simulink model "wlan_phy_rx_pmd"  (Simulink 7.8)
settings: Solver=VariableStepDiscrete  SolverMode=SingleTasking  StartTime=0.0  StopTime=simtime  RelTol=1e-3
PostLoadFcn:
  | wlan_phy_rx_init
InitFcn:
  | wlan_phy_rx_init
StartFcn:
  | wlan_phy_rx_init
values from neighbouring files (symbols this model uses):
  barker_seq20 = 0.95 * circshift(barker_seq20, [0 4]) ./ max(abs(barker_seq20))    [wlan_phy_rx_init.m]
  bit_scrambler_lfsr_bytes = bi2de(reshape(repmat(scr, 1, 8), 8, 127)', 'left-msb')    [wlan_phy_rx_init.m]
  deinterleave_ROM = [deinterleave_ROM interleave_64QAM zeros(1, 512-length(interleave_64QAM))]    [wlan_phy_rx_init.m]
  longCorr_coef_i = [3*real(LTS_corr)]    [wlan_phy_rx_init.m]
  longCorr_coef_q = [3*imag(LTS_corr)]    [wlan_phy_rx_init.m]
  MAX_CP_LEN = 32    [wlan_phy_rx_init.m]
  MAX_NCBPS = 288    [wlan_phy_rx_init.m]
  MAX_NUM_BYTES = 4096    [wlan_phy_rx_init.m]
  MAX_NUM_SC = 64    [wlan_phy_rx_init.m]
  MAX_NUM_SYMS = 600    [wlan_phy_rx_init.m]
  n_bits_preFFT_sampBuff = ceil(log2(4*MAX_NUM_SC))    [wlan_phy_rx_init.m]
  NCBPS_16QAM = 192    [wlan_phy_rx_init.m]
  NCBPS_64QAM = 288    [wlan_phy_rx_init.m]
  NCBPS_BPSK = 48    [wlan_phy_rx_init.m]
  NCBPS_QPSK = 96    [wlan_phy_rx_init.m]
  NDBPS_16QAM12 = 96    [wlan_phy_rx_init.m]
  NDBPS_16QAM34 = 144    [wlan_phy_rx_init.m]
  NDBPS_64QAM23 = 192    [wlan_phy_rx_init.m]
  NDBPS_64QAM34 = 216    [wlan_phy_rx_init.m]
  NDBPS_BPSK12 = 24    [wlan_phy_rx_init.m]
  NDBPS_BPSK34 = 36    [wlan_phy_rx_init.m]
  NDBPS_QPSK12 = 48    [wlan_phy_rx_init.m]
  NDBPS_QPSK34 = 72    [wlan_phy_rx_init.m]
  paylod_vec_samp_time = 8    [wlan_phy_rx_init.m]
  PHY_MIN_PKT_LEN = 14    [wlan_phy_rx_init.m]
  scr_ind_rev = zeros(1,128)    [wlan_phy_rx_init.m]
undefined symbols (not defined in the file or in its folder): gcbh, xlBlockLoadCallback, xlGetNormalizedPeriod, xlipgetpartsetting
libraries: xbsIndex_r4 x3519, simulink x1, xbsEDKLib_r4 x1
(part 6 of 21)

== system wlan_phy_rx_pmd/Inputs/RF A Inputs ==
  Rst : Inport
  IQ Valid : Inport  Port=2
  Constant2 : Constant  Value=2
  Constant4 : Constant  Value=5
  Delay1 : xbsIndex_r4/Delay  rst=off  infoeditControl=Selection of Reset will increase slice count due to use of real FFs and instead of SRLs  en=off  latency=1  dbl_ovrd=off  reg_retiming=off  block_type=delay
  Delay2 : xbsIndex_r4/Delay  rst=off  infoeditControl=Selection of Reset will increase slice count due to use of real FFs and instead of SRLs  en=off  latency=1  dbl_ovrd=off  reg_retiming=off  block_type=delay
  Down Sample : xbsIndex_r4/Down Sample  sample_ratio=16  sample_phase="Last Value of Frame  (most efficient)"  en=off  latency=1  dbl_ovrd=off  block_type=dsamp
  From Workspace : FromWorkspace  VariableName=raw_rx_I  SampleTime=paylod_vec_samp_time  Interpolate=off  OutputAfterFinalValue=Setting to zero
  From Workspace1 : FromWorkspace  VariableName=raw_rx_Q  SampleTime=paylod_vec_samp_time  Interpolate=off  OutputAfterFinalValue=Setting to zero
  From Workspace2 : FromWorkspace  VariableName=samps_rssi_avg  SampleTime=paylod_vec_samp_time  Interpolate=off  OutputAfterFinalValue=Setting to zero
  RF A RSSI Sum : Subsystem
  RFA_G_BB : xbsIndex_r4/Gateway In  gui_display_data_type=Fixed-point  arith_type=Unsigned  n_bits=5  bin_pt=0  preci_type=Single  exp_width=8  frac_width=24  quantization="Round  (unbiased: +/- Inf)"  overflow=Saturate  period=1  dbl_ovrd=off  timing_constraint=None  locs_specified=off  LOCs={}  UseAsADC=off  ADCChannel='1'  inherit_from_input=off  hdl_port=on  block_type=gatewayin
  RFA_G_RF : xbsIndex_r4/Gateway In  gui_display_data_type=Fixed-point  arith_type=Unsigned  n_bits=2  bin_pt=0  preci_type=Single  exp_width=8  frac_width=24  quantization="Round  (unbiased: +/- Inf)"  overflow=Saturate  period=1  dbl_ovrd=off  timing_constraint=None  locs_specified=off  LOCs={}  UseAsADC=off  ADCChannel='1'  inherit_from_input=off  hdl_port=on  block_type=gatewayin
  RFA_RSSI : xbsIndex_r4/Gateway In  gui_display_data_type=Fixed-point  arith_type=Unsigned  n_bits=10  bin_pt=0  preci_type=Single  exp_width=8  frac_width=24  quantization="Round  (unbiased: +/- Inf)"  overflow=Saturate  period=1  dbl_ovrd=off  timing_constraint=None  locs_specified=off  LOCs={}  UseAsADC=off  ADCChannel='1'  inherit_from_input=off  hdl_port=on  block_type=gatewayin
  RFA_RX_I : xbsIndex_r4/Gateway In  gui_display_data_type=Fixed-point  arith_type="Signed  (2's comp)"  n_bits=16  bin_pt=15  preci_type=Single  exp_width=8  frac_width=24  quantization="Round  (unbiased: +/- Inf)"  overflow=Saturate  period=1  dbl_ovrd=off  timing_constraint=None  locs_specified=off  LOCs={}  UseAsADC=off  ADCChannel='1'  inherit_from_input=off  hdl_port=on  block_type=gatewayin
  RFA_RX_Q : xbsIndex_r4/Gateway In  gui_display_data_type=Fixed-point  arith_type="Signed  (2's comp)"  n_bits=16  bin_pt=15  preci_type=Single  exp_width=8  frac_width=24  quantization="Round  (unbiased: +/- Inf)"  overflow=Saturate  period=1  dbl_ovrd=off  timing_constraint=None  locs_specified=off  LOCs={}  UseAsADC=off  ADCChannel='1'  inherit_from_input=off  hdl_port=on  block_type=gatewayin
  Register : xbsIndex_r4/Register  init=0  rst=on  en=on  dbl_ovrd=off  block_type=register
  Register1 : xbsIndex_r4/Register  init=0  rst=on  en=on  dbl_ovrd=off  block_type=register
  Register2 : xbsIndex_r4/Register  init=0  rst=off  en=off  dbl_ovrd=off  block_type=register
  Register3 : xbsIndex_r4/Register  init=0  rst=on  en=off  dbl_ovrd=off  block_type=register
  Register4 : xbsIndex_r4/Register  init=0  rst=off  en=off  dbl_ovrd=off  block_type=register
  Register5 : xbsIndex_r4/Register  init=0  rst=off  en=off  dbl_ovrd=off  block_type=register
  ADC I : Outport
  ADC Q : Outport  Port=2
  Avg RSSI : Outport  Port=3
  tag scope: CS_ADC_I=global, CS_ADC_Q=global, CS_G_BB=global, CS_G_RF=global, CS_RFA_RSSI=global, RFA_AGC_G_BB=global, RFA_AGC_G_RF=global
  wires:
  From Workspace/1 -> RFA_RX_I/1
  From Workspace1/1 -> RFA_RX_Q/1
  RFA_RX_I/1 -> Register2/1
  RFA_RX_Q/1 -> Register4/1
  Register/1 -> ADC I/1
  Register1/1 -> ADC Q/1
  Register3/1 -> Down Sample/1
  Down Sample/1 -> RF A RSSI Sum/1(RSSI)
  RF A RSSI Sum/1(Sum) -> [CS_RFA_RSSI], Avg RSSI/1
  RFA_RSSI/1 -> Register5/1
  Register2/1 -> Register/1, [CS_ADC_I]
  Register4/1 -> Register1/1, [CS_ADC_Q]
  Register5/1 -> Register3/1
  Rst/1 -> Register/2, Register1/2, Register3/2
  IQ Valid/1 -> Register1/3, Register/3
  RFA_G_RF/1 -> Delay1/1
  RFA_G_BB/1 -> Delay2/1
  Delay1/1 -> [CS_G_RF], [RFA_AGC_G_RF]
  Delay2/1 -> [CS_G_BB], [RFA_AGC_G_BB]
  Constant2/1 -> RFA_G_RF/1
  Constant4/1 -> RFA_G_BB/1

== system wlan_phy_rx_pmd/Inputs/RF B Inputs ==
  Rst : Inport
  IQ Valid : Inport  Port=2
  Constant6 : Constant  Value=2
  Constant7 : Constant  Value=5
  Delay4 : xbsIndex_r4/Delay  rst=off  infoeditControl=Selection of Reset will increase slice count due to use of real FFs and instead of SRLs  en=off  latency=1  dbl_ovrd=off  reg_retiming=off  block_type=delay
  Delay5 : xbsIndex_r4/Delay  rst=off  infoeditControl=Selection of Reset will increase slice count due to use of real FFs and instead of SRLs  en=off  latency=1  dbl_ovrd=off  reg_retiming=off  block_type=delay
  Down Sample : xbsIndex_r4/Down Sample  sample_ratio=16  sample_phase="Last Value of Frame  (most efficient)"  en=off  latency=1  dbl_ovrd=off  block_type=dsamp
  From Workspace : FromWorkspace  VariableName=raw_rx_I  SampleTime=paylod_vec_samp_time  Interpolate=off  OutputAfterFinalValue=Setting to zero
  From Workspace1 : FromWorkspace  VariableName=raw_rx_Q  SampleTime=paylod_vec_samp_time  Interpolate=off  OutputAfterFinalValue=Setting to zero
  From Workspace2 : FromWorkspace  VariableName=samps_rssi_avg  SampleTime=paylod_vec_samp_time  Interpolate=off  OutputAfterFinalValue=Setting to zero
  RF B RSSI Sum : Subsystem
  RFB_G_BB : xbsIndex_r4/Gateway In  gui_display_data_type=Fixed-point  arith_type=Unsigned  n_bits=5  bin_pt=0  preci_type=Single  exp_width=8  frac_width=24  quantization="Round  (unbiased: +/- Inf)"  overflow=Saturate  period=1  dbl_ovrd=off  timing_constraint=None  locs_specified=off  LOCs={}  UseAsADC=off  ADCChannel='1'  inherit_from_input=off  hdl_port=on  block_type=gatewayin
  RFB_G_RF : xbsIndex_r4/Gateway In  gui_display_data_type=Fixed-point  arith_type=Unsigned  n_bits=2  bin_pt=0  preci_type=Single  exp_width=8  frac_width=24  quantization="Round  (unbiased: +/- Inf)"  overflow=Saturate  period=1  dbl_ovrd=off  timing_constraint=None  locs_specified=off  LOCs={}  UseAsADC=off  ADCChannel='1'  inherit_from_input=off  hdl_port=on  block_type=gatewayin
  RFB_RSSI : xbsIndex_r4/Gateway In  gui_display_data_type=Fixed-point  arith_type=Unsigned  n_bits=10  bin_pt=0  preci_type=Single  exp_width=8  frac_width=24  quantization="Round  (unbiased: +/- Inf)"  overflow=Saturate  period=1  dbl_ovrd=off  timing_constraint=None  locs_specified=off  LOCs={}  UseAsADC=off  ADCChannel='1'  inherit_from_input=off  hdl_port=on  block_type=gatewayin
  RFB_RX_I : xbsIndex_r4/Gateway In  gui_display_data_type=Fixed-point  arith_type="Signed  (2's comp)"  n_bits=16  bin_pt=15  preci_type=Single  exp_width=8  frac_width=24  quantization="Round  (unbiased: +/- Inf)"  overflow=Saturate  period=1  dbl_ovrd=off  timing_constraint=None  locs_specified=off  LOCs={}  UseAsADC=off  ADCChannel='1'  inherit_from_input=off  hdl_port=on  block_type=gatewayin
  RFB_RX_Q : xbsIndex_r4/Gateway In  gui_display_data_type=Fixed-point  arith_type="Signed  (2's comp)"  n_bits=16  bin_pt=15  preci_type=Single  exp_width=8  frac_width=24  quantization="Round  (unbiased: +/- Inf)"  overflow=Saturate  period=1  dbl_ovrd=off  timing_constraint=None  locs_specified=off  LOCs={}  UseAsADC=off  ADCChannel='1'  inherit_from_input=off  hdl_port=on  block_type=gatewayin
  Register : xbsIndex_r4/Register  init=0  rst=on  en=on  dbl_ovrd=off  block_type=register
  Register1 : xbsIndex_r4/Register  init=0  rst=on  en=on  dbl_ovrd=off  block_type=register
  Register2 : xbsIndex_r4/Register  init=0  rst=off  en=off  dbl_ovrd=off  block_type=register
  Register3 : xbsIndex_r4/Register  init=0  rst=on  en=off  dbl_ovrd=off  block_type=register
  Register4 : xbsIndex_r4/Register  init=0  rst=off  en=off  dbl_ovrd=off  block_type=register
  Register5 : xbsIndex_r4/Register  init=0  rst=off  en=off  dbl_ovrd=off  block_type=register
  ADC I : Outport
  ADC Q : Outport  Port=2
  Avg RSSI : Outport  Port=3
  tag scope: RFB_AGC_G_BB=global, RFB_AGC_G_RF=global
  wires:
  IQ Valid/1 -> Register/3, Register1/3
  Rst/1 -> Register3/2, Register1/2, Register/2
  Register5/1 -> Register3/1
  Register4/1 -> Register1/1
  Register2/1 -> Register/1
  RFB_RSSI/1 -> Register5/1
  RF B RSSI Sum/1(Sum) -> Avg RSSI/1
  Down Sample/1 -> RF B RSSI Sum/1(RSSI)
  Register3/1 -> Down Sample/1
  Register1/1 -> ADC Q/1
  Register/1 -> ADC I/1
  RFB_RX_Q/1 -> Register4/1
  RFB_RX_I/1 -> Register2/1
  RFB_G_RF/1 -> Delay4/1
  RFB_G_BB/1 -> Delay5/1
  Delay4/1 -> [RFB_AGC_G_RF]
  Delay5/1 -> [RFB_AGC_G_BB]
  Constant6/1 -> RFB_G_RF/1
  Constant7/1 -> RFB_G_BB/1

== system wlan_phy_rx_pmd/Inputs/RF C Inputs ==
  Rst : Inport
  IQ Valid : Inport  Port=2
  Constant2 : Constant  Value=2
  Constant4 : Constant  Value=5
  Delay1 : xbsIndex_r4/Delay  rst=off  infoeditControl=Selection of Reset will increase slice count due to use of real FFs and instead of SRLs  en=off  latency=1  dbl_ovrd=off  reg_retiming=off  block_type=delay
  Delay2 : xbsIndex_r4/Delay  rst=off  infoeditControl=Selection of Reset will increase slice count due to use of real FFs and instead of SRLs  en=off  latency=1  dbl_ovrd=off  reg_retiming=off  block_type=delay
  Down Sample : xbsIndex_r4/Down Sample  sample_ratio=16  sample_phase="Last Value of Frame  (most efficient)"  en=off  latency=1  dbl_ovrd=off  block_type=dsamp
  From Workspace : FromWorkspace  VariableName=raw_rx_I  SampleTime=paylod_vec_samp_time  Interpolate=off  OutputAfterFinalValue=Setting to zero
  From Workspace1 : FromWorkspace  VariableName=raw_rx_Q  SampleTime=paylod_vec_samp_time  Interpolate=off  OutputAfterFinalValue=Setting to zero
  From Workspace2 : FromWorkspace  VariableName=samps_rssi_avg  SampleTime=paylod_vec_samp_time  Interpolate=off  OutputAfterFinalValue=Setting to zero
  RF C RSSI Sum : Subsystem
  RFC_G_BB : xbsIndex_r4/Gateway In  gui_display_data_type=Fixed-point  arith_type=Unsigned  n_bits=5  bin_pt=0  preci_type=Single  exp_width=8  frac_width=24  quantization="Round  (unbiased: +/- Inf)"  overflow=Saturate  period=1  dbl_ovrd=off  timing_constraint=None  locs_specified=off  LOCs={}  UseAsADC=off  ADCChannel='1'  inherit_from_input=off  hdl_port=on  block_type=gatewayin
  RFC_G_RF : xbsIndex_r4/Gateway In  gui_display_data_type=Fixed-point  arith_type=Unsigned  n_bits=2  bin_pt=0  preci_type=Single  exp_width=8  frac_width=24  quantization="Round  (unbiased: +/- Inf)"  overflow=Saturate  period=1  dbl_ovrd=off  timing_constraint=None  locs_specified=off  LOCs={}  UseAsADC=off  ADCChannel='1'  inherit_from_input=off  hdl_port=on  block_type=gatewayin
  RFC_RSSI : xbsIndex_r4/Gateway In  gui_display_data_type=Fixed-point  arith_type=Unsigned  n_bits=10  bin_pt=0  preci_type=Single  exp_width=8  frac_width=24  quantization="Round  (unbiased: +/- Inf)"  overflow=Saturate  period=1  dbl_ovrd=off  timing_constraint=None  locs_specified=off  LOCs={}  UseAsADC=off  ADCChannel='1'  inherit_from_input=off  hdl_port=on  block_type=gatewayin
  RFC_RX_I : xbsIndex_r4/Gateway In  gui_display_data_type=Fixed-point  arith_type="Signed  (2's comp)"  n_bits=16  bin_pt=15  preci_type=Single  exp_width=8  frac_width=24  quantization="Round  (unbiased: +/- Inf)"  overflow=Saturate  period=1  dbl_ovrd=off  timing_constraint=None  locs_specified=off  LOCs={}  UseAsADC=off  ADCChannel='1'  inherit_from_input=off  hdl_port=on  block_type=gatewayin
  RFC_RX_Q : xbsIndex_r4/Gateway In  gui_display_data_type=Fixed-point  arith_type="Signed  (2's comp)"  n_bits=16  bin_pt=15  preci_type=Single  exp_width=8  frac_width=24  quantization="Round  (unbiased: +/- Inf)"  overflow=Saturate  period=1  dbl_ovrd=off  timing_constraint=None  locs_specified=off  LOCs={}  UseAsADC=off  ADCChannel='1'  inherit_from_input=off  hdl_port=on  block_type=gatewayin
  Register : xbsIndex_r4/Register  init=0  rst=on  en=on  dbl_ovrd=off  block_type=register
  Register1 : xbsIndex_r4/Register  init=0  rst=on  en=on  dbl_ovrd=off  block_type=register
  Register2 : xbsIndex_r4/Register  init=0  rst=off  en=off  dbl_ovrd=off  block_type=register
  Register3 : xbsIndex_r4/Register  init=0  rst=on  en=off  dbl_ovrd=off  block_type=register
  Register4 : xbsIndex_r4/Register  init=0  rst=off  en=off  dbl_ovrd=off  block_type=register
  Register5 : xbsIndex_r4/Register  init=0  rst=off  en=off  dbl_ovrd=off  block_type=register
  ADC I : Outport
  ADC Q : Outport  Port=2
  Avg RSSI : Outport  Port=3
  tag scope: CS_ADC_I_RFC=global, CS_ADC_Q_RFC=global, RFC_AGC_G_BB=global, RFC_AGC_G_RF=global
  wires:
  Constant4/1 -> RFC_G_BB/1
  Constant2/1 -> RFC_G_RF/1
  Delay2/1 -> [RFC_AGC_G_BB]
  Delay1/1 -> [RFC_AGC_G_RF]
  RFC_G_BB/1 -> Delay2/1
  RFC_G_RF/1 -> Delay1/1
  IQ Valid/1 -> Register/3, Register1/3
  Rst/1 -> Register3/2, Register1/2, Register/2
  Register5/1 -> Register3/1
  Register4/1 -> Register1/1, [CS_ADC_Q_RFC]
  Register2/1 -> Register/1, [CS_ADC_I_RFC]
  RFC_RSSI/1 -> Register5/1
  RF C RSSI Sum/1(Sum) -> Avg RSSI/1
  Down Sample/1 -> RF C RSSI Sum/1(RSSI)
  Register3/1 -> Down Sample/1
  Register1/1 -> ADC Q/1
  Register/1 -> ADC I/1
  RFC_RX_Q/1 -> Register4/1
  RFC_RX_I/1 -> Register2/1
  From Workspace1/1 -> RFC_RX_Q/1
  From Workspace/1 -> RFC_RX_I/1

== system wlan_phy_rx_pmd/Inputs/RF D Inputs ==
  Rst : Inport
  IQ Valid : Inport  Port=2
  Constant6 : Constant  Value=2
  Constant7 : Constant  Value=5
  Delay4 : xbsIndex_r4/Delay  rst=off  infoeditControl=Selection of Reset will increase slice count due to use of real FFs and instead of SRLs  en=off  latency=1  dbl_ovrd=off  reg_retiming=off  block_type=delay
  Delay5 : xbsIndex_r4/Delay  rst=off  infoeditControl=Selection of Reset will increase slice count due to use of real FFs and instead of SRLs  en=off  latency=1  dbl_ovrd=off  reg_retiming=off  block_type=delay
  Down Sample : xbsIndex_r4/Down Sample  sample_ratio=16  sample_phase="Last Value of Frame  (most efficient)"  en=off  latency=1  dbl_ovrd=off  block_type=dsamp
  From Workspace : FromWorkspace  VariableName=raw_rx_I  SampleTime=paylod_vec_samp_time  Interpolate=off  OutputAfterFinalValue=Setting to zero
  From Workspace1 : FromWorkspace  VariableName=raw_rx_Q  SampleTime=paylod_vec_samp_time  Interpolate=off  OutputAfterFinalValue=Setting to zero
  From Workspace2 : FromWorkspace  VariableName=samps_rssi_avg  SampleTime=paylod_vec_samp_time  Interpolate=off  OutputAfterFinalValue=Setting to zero
  RF D RSSI Sum : Subsystem
  RFD_G_BB : xbsIndex_r4/Gateway In  gui_display_data_type=Fixed-point  arith_type=Unsigned  n_bits=5  bin_pt=0  preci_type=Single  exp_width=8  frac_width=24  quantization="Round  (unbiased: +/- Inf)"  overflow=Saturate  period=1  dbl_ovrd=off  timing_constraint=None  locs_specified=off  LOCs={}  UseAsADC=off  ADCChannel='1'  inherit_from_input=off  hdl_port=on  block_type=gatewayin
  RFD_G_RF : xbsIndex_r4/Gateway In  gui_display_data_type=Fixed-point  arith_type=Unsigned  n_bits=2  bin_pt=0  preci_type=Single  exp_width=8  frac_width=24  quantization="Round  (unbiased: +/- Inf)"  overflow=Saturate  period=1  dbl_ovrd=off  timing_constraint=None  locs_specified=off  LOCs={}  UseAsADC=off  ADCChannel='1'  inherit_from_input=off  hdl_port=on  block_type=gatewayin
  RFD_RSSI : xbsIndex_r4/Gateway In  gui_display_data_type=Fixed-point  arith_type=Unsigned  n_bits=10  bin_pt=0  preci_type=Single  exp_width=8  frac_width=24  quantization="Round  (unbiased: +/- Inf)"  overflow=Saturate  period=1  dbl_ovrd=off  timing_constraint=None  locs_specified=off  LOCs={}  UseAsADC=off  ADCChannel='1'  inherit_from_input=off  hdl_port=on  block_type=gatewayin
  RFD_RX_I : xbsIndex_r4/Gateway In  gui_display_data_type=Fixed-point  arith_type="Signed  (2's comp)"  n_bits=16  bin_pt=15  preci_type=Single  exp_width=8  frac_width=24  quantization="Round  (unbiased: +/- Inf)"  overflow=Saturate  period=1  dbl_ovrd=off  timing_constraint=None  locs_specified=off  LOCs={}  UseAsADC=off  ADCChannel='1'  inherit_from_input=off  hdl_port=on  block_type=gatewayin
  RFD_RX_Q : xbsIndex_r4/Gateway In  gui_display_data_type=Fixed-point  arith_type="Signed  (2's comp)"  n_bits=16  bin_pt=15  preci_type=Single  exp_width=8  frac_width=24  quantization="Round  (unbiased: +/- Inf)"  overflow=Saturate  period=1  dbl_ovrd=off  timing_constraint=None  locs_specified=off  LOCs={}  UseAsADC=off  ADCChannel='1'  inherit_from_input=off  hdl_port=on  block_type=gatewayin
  Register : xbsIndex_r4/Register  init=0  rst=on  en=on  dbl_ovrd=off  block_type=register
  Register1 : xbsIndex_r4/Register  init=0  rst=on  en=on  dbl_ovrd=off  block_type=register
  Register2 : xbsIndex_r4/Register  init=0  rst=off  en=off  dbl_ovrd=off  block_type=register
  Register3 : xbsIndex_r4/Register  init=0  rst=on  en=off  dbl_ovrd=off  block_type=register
  Register4 : xbsIndex_r4/Register  init=0  rst=off  en=off  dbl_ovrd=off  block_type=register
  Register5 : xbsIndex_r4/Register  init=0  rst=off  en=off  dbl_ovrd=off  block_type=register
  ADC I : Outport
  ADC Q : Outport  Port=2
  Avg RSSI : Outport  Port=3
  tag scope: RFD_AGC_G_BB=global, RFD_AGC_G_RF=global
  wires:
  Constant7/1 -> RFD_G_BB/1
  Constant6/1 -> RFD_G_RF/1
  Delay5/1 -> [RFD_AGC_G_BB]
  Delay4/1 -> [RFD_AGC_G_RF]
  RFD_G_BB/1 -> Delay5/1
  RFD_G_RF/1 -> Delay4/1
  RFD_RX_I/1 -> Register2/1
  RFD_RX_Q/1 -> Register4/1
  Register/1 -> ADC I/1
  Register1/1 -> ADC Q/1
  Register3/1 -> Down Sample/1
  Down Sample/1 -> RF D RSSI Sum/1(RSSI)
  RF D RSSI Sum/1(Sum) -> Avg RSSI/1
  RFD_RSSI/1 -> Register5/1
  Register2/1 -> Register/1
  Register4/1 -> Register1/1
  Register5/1 -> Register3/1
  Rst/1 -> Register/2, Register1/2, Register3/2
  IQ Valid/1 -> Register1/3, Register/3

== system wlan_phy_rx_pmd/Inputs/RSSI Clock Gen ==
  1LSB+3 : xbsIndex_r4/Slice  nbits=1  boolean_output=off  mode=Lower Bit Location + Width  bit1=0  base1=MSB of Input  bit0=3  base0=LSB of Input  dbl_ovrd=off  block_type=slice
  RSSI Clock Generator : xbsIndex_r4/Counter  cnt_type=Free Running  cnt_to=Inf  operation=Up  start_count=0  cnt_by_val=1  arith_type=Unsigned  n_bits=4  bin_pt=0  load_pin=off  rst=off  en=off  explicit_period=on  period=1  dbl_ovrd=off  use_behavioral_HDL=off  implementation=Fabric  use_rpm=off  block_type=counter
      settings: block_version=VER_STRING_GOES_HERE
  Clk : Outport
  wires:
  RSSI Clock Generator/1 -> 1LSB+3/1
  1LSB+3/1 -> Clk/1

== system wlan_phy_rx_pmd/Inputs/negedge1 ==
  D : Inport
  Delay : xbsIndex_r4/Delay  rst=off  infoeditControl=Selection of Reset will increase slice count due to use of real FFs and instead of SRLs  en=off  latency=1  dbl_ovrd=off  reg_retiming=off  block_type=delay
      settings: infoedit=Hardware notes: A delay line is a chain, each link of which is an SRL16 followed by a flip-flop. If register retiming is enabled, the delay line is a chain of flip-flops.  xl_area=[1 1 0 0 0 0 0]  block_version=VER_STRING_GOES_HERE
  Inverter : xbsIndex_r4/Inverter  en=off  latency=0  dbl_ovrd=off  block_type=inv
      settings: xl_area=[0 0 0 0 0 0 0]  block_version=VER_STRING_GOES_HERE
  Logical1 : xbsIndex_r4/Logical  logical_function=AND  inputs=2  en=off  latency=0  precision=Full  arith_type=Unsigned  n_bits=8  bin_pt=2  align_bp=on  dbl_ovrd=off  block_type=logical
      settings: xl_area=[1 0 0 1 0 0 0]  block_version=VER_STRING_GOES_HERE
  Q : Outport
  wires:
  D/1 -> Delay/1, Inverter/1
  Logical1/1 -> Q/1
  Delay/1 -> Logical1/1
  Inverter/1 -> Logical1/2

== system wlan_phy_rx_pmd/MAC Outputs/S-R Latch1 ==
  S : Inport
  R : Inport  Port=2
  Constant1 : xbsIndex_r4/Constant  const=1  gui_display_data_type=Fixed-point  arith_type=Boolean  n_bits=1  bin_pt=0  preci_type=Single  exp_width=8  frac_width=24  explicit_period=off  period=1  equ=P=C  opselect=C  inp2=PCIN>>17  opr=+  inp1=P  carry=CIN  dbl_ovrd=off  block_type=constant
      settings: block_version=VER_STRING_GOES_HERE
  Convert1 : xbsIndex_r4/Convert  gui_display_data_type=Boolean  arith_type=Boolean  n_bits=1  bin_pt=0  float_type=Single  exp_bits=8  fraction_bits=24  quantization=Truncate  overflow=Wrap  en=off  latency=0  dbl_ovrd=off  pipeline=off  block_type=convert
  Convert2 : xbsIndex_r4/Convert  gui_display_data_type=Boolean  arith_type=Boolean  n_bits=1  bin_pt=0  float_type=Single  exp_bits=8  fraction_bits=24  quantization=Truncate  overflow=Wrap  en=off  latency=0  dbl_ovrd=off  pipeline=off  block_type=convert
  Register2 : xbsIndex_r4/Register  init=0  rst=on  en=on  dbl_ovrd=off  block_type=register
      settings: xl_area=[1 1 0 0 0 0 0]  block_version=VER_STRING_GOES_HERE
  Q : Outport
  wires:
  Convert2/1 -> Register2/3
  Convert1/1 -> Register2/2
  Constant1/1 -> Register2/1
  R/1 -> Convert1/1
  Register2/1 -> Q/1
  S/1 -> Convert2/1

== system wlan_phy_rx_pmd/MAC Outputs/S-R Latch2 ==
  S : Inport
  R : Inport  Port=2
  Constant1 : xbsIndex_r4/Constant  const=1  gui_display_data_type=Fixed-point  arith_type=Boolean  n_bits=1  bin_pt=0  preci_type=Single  exp_width=8  frac_width=24  explicit_period=off  period=1  equ=P=C  opselect=C  inp2=PCIN>>17  opr=+  inp1=P  carry=CIN  dbl_ovrd=off  block_type=constant
      settings: block_version=VER_STRING_GOES_HERE
  Convert1 : xbsIndex_r4/Convert  gui_display_data_type=Boolean  arith_type=Boolean  n_bits=1  bin_pt=0  float_type=Single  exp_bits=8  fraction_bits=24  quantization=Truncate  overflow=Wrap  en=off  latency=0  dbl_ovrd=off  pipeline=off  block_type=convert
  Convert2 : xbsIndex_r4/Convert  gui_display_data_type=Boolean  arith_type=Boolean  n_bits=1  bin_pt=0  float_type=Single  exp_bits=8  fraction_bits=24  quantization=Truncate  overflow=Wrap  en=off  latency=0  dbl_ovrd=off  pipeline=off  block_type=convert
  Register2 : xbsIndex_r4/Register  init=0  rst=on  en=on  dbl_ovrd=off  block_type=register
      settings: xl_area=[1 1 0 0 0 0 0]  block_version=VER_STRING_GOES_HERE
  Q : Outport
  wires:
  S/1 -> Convert2/1
  Register2/1 -> Q/1
  R/1 -> Convert1/1
  Constant1/1 -> Register2/1
  Convert1/1 -> Register2/2
  Convert2/1 -> Register2/3

== system wlan_phy_rx_pmd/Registers/2x  ==
  d : Inport
  Concat2 : xbsIndex_r4/Concat  num_inputs=2  dbl_ovrd=off  block_type=concat
      settings: block_version=8.2.02
  Constant2 : xbsIndex_r4/Constant  const=0  gui_display_data_type=Fixed-point  arith_type=Unsigned  n_bits=1  bin_pt=0  preci_type=Single  exp_width=8  frac_width=24  explicit_period=on  period=1  equ=P=C  opselect=C  inp2=PCIN>>17  opr=+  inp1=P  carry=CIN  dbl_ovrd=off  block_type=constant
  2d : Outport
  wires:
  Concat2/1 -> 2d/1
  d/1 -> Concat2/1
  Constant2/1 -> Concat2/2

== system wlan_phy_rx_pmd/Registers/Config Bits ==
  32b : Inport
  "b[0]   " : xbsIndex_r4/Slice  nbits=1  boolean_output=on  mode=Lower Bit Location + Width  bit1=0  base1=MSB of Input  bit0=0  base0=LSB of Input  dbl_ovrd=off  block_type=slice
      settings: block_version=VER_STRING_GOES_HERE
  b[10] : xbsIndex_r4/Slice  nbits=1  boolean_output=on  mode=Lower Bit Location + Width  bit1=0  base1=MSB of Input  bit0=10  base0=LSB of Input  dbl_ovrd=off  block_type=slice
      settings: block_version=VER_STRING_GOES_HERE
  b[11] : xbsIndex_r4/Slice  nbits=1  boolean_output=on  mode=Lower Bit Location + Width  bit1=0  base1=MSB of Input  bit0=11  base0=LSB of Input  dbl_ovrd=off  block_type=slice
      settings: block_version=VER_STRING_GOES_HERE
  b[12] : xbsIndex_r4/Slice  nbits=1  boolean_output=on  mode=Lower Bit Location + Width  bit1=0  base1=MSB of Input  bit0=12  base0=LSB of Input  dbl_ovrd=off  block_type=slice
      settings: block_version=VER_STRING_GOES_HERE
  b[13] : xbsIndex_r4/Slice  nbits=1  boolean_output=on  mode=Lower Bit Location + Width  bit1=0  base1=MSB of Input  bit0=13  base0=LSB of Input  dbl_ovrd=off  block_type=slice
      settings: block_version=VER_STRING_GOES_HERE
  b[14] : xbsIndex_r4/Slice  nbits=1  boolean_output=on  mode=Lower Bit Location + Width  bit1=0  base1=MSB of Input  bit0=14  base0=LSB of Input  dbl_ovrd=off  block_type=slice
      settings: block_version=VER_STRING_GOES_HERE
  b[16:15] : xbsIndex_r4/Slice  nbits=2  boolean_output=off  mode=Lower Bit Location + Width  bit1=0  base1=MSB of Input  bit0=15  base0=LSB of Input  dbl_ovrd=off  block_type=slice
      settings: block_version=VER_STRING_GOES_HERE
  "b[1]  " : xbsIndex_r4/Slice  nbits=1  boolean_output=on  mode=Lower Bit Location + Width  bit1=0  base1=MSB of Input  bit0=1  base0=LSB of Input  dbl_ovrd=off  block_type=slice
      settings: block_version=VER_STRING_GOES_HERE
  b[20:17] : xbsIndex_r4/Slice  nbits=4  boolean_output=off  mode=Lower Bit Location + Width  bit1=0  base1=MSB of Input  bit0=17  base0=LSB of Input  dbl_ovrd=off  block_type=slice
      settings: block_version=VER_STRING_GOES_HERE
  b[2] : xbsIndex_r4/Slice  nbits=1  boolean_output=off  mode=Lower Bit Location + Width  bit1=0  base1=MSB of Input  bit0=2  base0=LSB of Input  dbl_ovrd=off  block_type=slice
      settings: block_version=VER_STRING_GOES_HERE
  b[3] : xbsIndex_r4/Slice  nbits=1  boolean_output=off  mode=Lower Bit Location + Width  bit1=0  base1=MSB of Input  bit0=3  base0=LSB of Input  dbl_ovrd=off  block_type=slice
      settings: block_version=VER_STRING_GOES_HERE
  b[4] : xbsIndex_r4/Slice  nbits=1  boolean_output=off  mode=Lower Bit Location + Width  bit1=0  base1=MSB of Input  bit0=4  base0=LSB of Input  dbl_ovrd=off  block_type=slice
      settings: block_version=VER_STRING_GOES_HERE
  b[5] : xbsIndex_r4/Slice  nbits=1  boolean_output=on  mode=Lower Bit Location + Width  bit1=0  base1=MSB of Input  bit0=5  base0=LSB of Input  dbl_ovrd=off  block_type=slice
      settings: block_version=VER_STRING_GOES_HERE
  b[6] : xbsIndex_r4/Slice  nbits=1  boolean_output=on  mode=Lower Bit Location + Width  bit1=0  base1=MSB of Input  bit0=6  base0=LSB of Input  dbl_ovrd=off  block_type=slice
      settings: block_version=VER_STRING_GOES_HERE
  b[7] : xbsIndex_r4/Slice  nbits=1  boolean_output=on  mode=Lower Bit Location + Width  bit1=0  base1=MSB of Input  bit0=7  base0=LSB of Input  dbl_ovrd=off  block_type=slice
      settings: block_version=VER_STRING_GOES_HERE
  b[8] : xbsIndex_r4/Slice  nbits=1  boolean_output=on  mode=Lower Bit Location + Width  bit1=0  base1=MSB of Input  bit0=8  base0=LSB of Input  dbl_ovrd=off  block_type=slice
      settings: block_version=VER_STRING_GOES_HERE
  b[9] : xbsIndex_r4/Slice  nbits=1  boolean_output=on  mode=Lower Bit Location + Width  bit1=0  base1=MSB of Input  bit0=9  base0=LSB of Input  dbl_ovrd=off  block_type=slice
      settings: block_version=VER_STRING_GOES_HERE
  tag scope: REG_USE_RX_SIGS_INVALID_INPUT=global, regRx_BYPASS_CFO_EST=global, regRx_DSSS_RX_EN=global, regRx_DSSS_RX_PKT_DET_OUT_EN=global, regRx_DSSS_RX_REQ_AGC_DONE=global, regRx_MAX_SIGNAL_LENGTH_KB=global, regRx_PKT_BUF_BYTE_ORDER_SWAP=global, regRx_PKT_BUF_H_EST_ORDER_SWAP=global, regRx_Record_Chan_Est_Pkt_Buf=global, regRx_noSwitching_AntSel=global, regRx_phyCCA_Mode_Sel=global, regRx_pktDetEn_Ext=global, regRx_pktDetEn_RFA=global, regRx_pktDetEn_RFB=global, regRx_pktDetEn_RFC=global, regRx_pktDetEn_RFD=global, regRx_switchingDiv_En=global
  wires:
  32b/1 -> "b[1]  "/1, b[2]/1, b[3]/1, b[4]/1, b[5]/1, b[6]/1, b[7]/1, b[9]/1, b[10]/1, b[12]/1, b[13]/1, b[14]/1, b[16:15]/1, b[20:17]/1, b[11]/1, b[8]/1, "b[0]   "/1
  "b[0]   "/1 -> [regRx_DSSS_RX_EN]
  "b[1]  "/1 -> [REG_USE_RX_SIGS_INVALID_INPUT]
  b[2]/1 -> [regRx_PKT_BUF_BYTE_ORDER_SWAP]
  b[3]/1 -> [regRx_PKT_BUF_H_EST_ORDER_SWAP]
  b[4]/1 -> [regRx_DSSS_RX_PKT_DET_OUT_EN]
  b[5]/1 -> [regRx_BYPASS_CFO_EST]
  b[6]/1 -> [regRx_Record_Chan_Est_Pkt_Buf]
  b[7]/1 -> [regRx_switchingDiv_En]
  b[16:15]/1 -> [regRx_noSwitching_AntSel]
  b[9]/1 -> [regRx_pktDetEn_RFA]
  b[10]/1 -> [regRx_pktDetEn_RFB]
  b[13]/1 -> [regRx_pktDetEn_Ext]
  b[11]/1 -> [regRx_pktDetEn_RFC]
  b[12]/1 -> [regRx_pktDetEn_RFD]
  b[14]/1 -> [regRx_phyCCA_Mode_Sel]
  b[8]/1 -> [regRx_DSSS_RX_REQ_AGC_DONE]
  b[20:17]/1 -> [regRx_MAX_SIGNAL_LENGTH_KB]

== system wlan_phy_rx_pmd/Registers/Fanout ==
  Corr_Thresh : Inport
  E_Thresh : Inport  Port=2
  MinDur : Inport  Port=3
  Register{1..11,20} (12 alike) : xbsIndex_r4/Register  init=0  rst=off  en=off  dbl_ovrd=off  block_type=register
  tag scope: regPktDet_pktDetMinDuration_autoCorr_A=global, regPktDet_pktDetMinDuration_autoCorr_B=global, regPktDet_pktDetMinDuration_autoCorr_C=global, regPktDet_pktDetMinDuration_autoCorr_D=global, regRx_PktDet_CorrThresh_A=global, regRx_PktDet_CorrThresh_B=global, regRx_PktDet_CorrThresh_C=global, regRx_PktDet_CorrThresh_D=global, regRx_PktDet_EnergyThresh_A=global, regRx_PktDet_EnergyThresh_B=global, regRx_PktDet_EnergyThresh_C=global, regRx_PktDet_EnergyThresh_D=global
  wires:
  Corr_Thresh/1 -> Register20/1, Register1/1, Register2/1, Register3/1
  E_Thresh/1 -> Register4/1, Register5/1, Register6/1, Register7/1
  MinDur/1 -> Register8/1, Register9/1, Register10/1, Register11/1
  Register20/1 -> [regRx_PktDet_CorrThresh_D]
  Register1/1 -> [regRx_PktDet_CorrThresh_C]
  Register2/1 -> [regRx_PktDet_CorrThresh_B]
  Register3/1 -> [regRx_PktDet_CorrThresh_A]
  Register4/1 -> [regRx_PktDet_EnergyThresh_A]
  Register5/1 -> [regRx_PktDet_EnergyThresh_B]
  Register6/1 -> [regRx_PktDet_EnergyThresh_C]
  Register7/1 -> [regRx_PktDet_EnergyThresh_D]
  Register8/1 -> [regPktDet_pktDetMinDuration_autoCorr_A]
  Register9/1 -> [regPktDet_pktDetMinDuration_autoCorr_B]
  Register10/1 -> [regPktDet_pktDetMinDuration_autoCorr_C]
  Register11/1 -> [regPktDet_pktDetMinDuration_autoCorr_D]

== system wlan_phy_rx_pmd/Registers/Fanout1 ==
  In1 : Inport
  In2 : Inport  Port=2
  In3 : Inport  Port=3
  Register{1..11,20} (12 alike) : xbsIndex_r4/Register  init=0  rst=off  en=off  dbl_ovrd=off  block_type=register
  tag scope: regRx_PKTDET_RSSI_MIN_DUR_A=global, regRx_PKTDET_RSSI_MIN_DUR_B=global, regRx_PKTDET_RSSI_MIN_DUR_C=global, regRx_PKTDET_RSSI_MIN_DUR_D=global, regRx_PKTDET_RSSI_THRESH_A=global, regRx_PKTDET_RSSI_THRESH_B=global, regRx_PKTDET_RSSI_THRESH_C=global, regRx_PKTDET_RSSI_THRESH_D=global, regRx_RSSI_SUM_LEN_A=global, regRx_RSSI_SUM_LEN_B=global, regRx_RSSI_SUM_LEN_C=global, regRx_RSSI_SUM_LEN_D=global
  wires:
  Register3/1 -> [regRx_RSSI_SUM_LEN_A]
  Register2/1 -> [regRx_RSSI_SUM_LEN_B]
  Register1/1 -> [regRx_RSSI_SUM_LEN_C]
  Register20/1 -> [regRx_RSSI_SUM_LEN_D]
  In1/1 -> Register3/1, Register2/1, Register1/1, Register20/1
  In2/1 -> Register6/1, Register4/1, Register5/1, Register7/1
  In3/1 -> Register11/1, Register9/1, Register8/1, Register10/1
  Register11/1 -> [regRx_PKTDET_RSSI_MIN_DUR_A]
  Register9/1 -> [regRx_PKTDET_RSSI_MIN_DUR_B]
  Register8/1 -> [regRx_PKTDET_RSSI_MIN_DUR_C]
  Register10/1 -> [regRx_PKTDET_RSSI_MIN_DUR_D]
  Register7/1 -> [regRx_PKTDET_RSSI_THRESH_A]
  Register5/1 -> [regRx_PKTDET_RSSI_THRESH_B]
  Register4/1 -> [regRx_PKTDET_RSSI_THRESH_C]
  Register6/1 -> [regRx_PKTDET_RSSI_THRESH_D]

== system wlan_phy_rx_pmd/Registers/Fanout2 ==
  Corr_Thresh : Inport
  E_Thresh : Inport  Port=2
  Register{1..7,20} (8 alike) : xbsIndex_r4/Register  init=0  rst=off  en=off  dbl_ovrd=off  block_type=register
  tag scope: regRx_PktDet_CorrThresh_DSSS_A=global, regRx_PktDet_CorrThresh_DSSS_B=global, regRx_PktDet_CorrThresh_DSSS_C=global, regRx_PktDet_CorrThresh_DSSS_D=global, regRx_PktDet_EnergyThresh_DSSS_A=global, regRx_PktDet_EnergyThresh_DSSS_B=global, regRx_PktDet_EnergyThresh_DSSS_C=global, regRx_PktDet_EnergyThresh_DSSS_D=global
  wires:
  Register3/1 -> [regRx_PktDet_CorrThresh_DSSS_A]
  Register2/1 -> [regRx_PktDet_CorrThresh_DSSS_B]
  Register1/1 -> [regRx_PktDet_CorrThresh_DSSS_C]
  Register20/1 -> [regRx_PktDet_CorrThresh_DSSS_D]
  Corr_Thresh/1 -> Register3/1, Register2/1, Register1/1, Register20/1
  E_Thresh/1 -> Register4/1, Register5/1, Register6/1, Register7/1
  Register4/1 -> [regRx_PktDet_EnergyThresh_DSSS_A]
  Register5/1 -> [regRx_PktDet_EnergyThresh_DSSS_B]
  Register6/1 -> [regRx_PktDet_EnergyThresh_DSSS_C]
  Register7/1 -> [regRx_PktDet_EnergyThresh_DSSS_D]

== system wlan_phy_rx_pmd/Registers/Sim Reset ==
  Add : Sum
  Disregard Subsystem : xbsIndex_r4/Disregard Subsystem  Tag=discardX  block_type=disregard
  Pulse Generator : DiscretePulseGenerator  Period=1e6  PulseWidth=2  PhaseDelay=1.25e4
  Pulse Generator1 : DiscretePulseGenerator  Period=1e6  PulseWidth=2  PhaseDelay=2.5e4
  sim_only_reset : xbsIndex_r4/Gateway In  gui_display_data_type=Fixed-point  arith_type=Unsigned  n_bits=1  bin_pt=0  preci_type=Single  exp_width=8  frac_width=24  quantization="Round  (unbiased: +/- Inf)"  overflow=Saturate  period=1  dbl_ovrd=off  timing_constraint=None  locs_specified=off  LOCs={}  UseAsADC=off  ADCChannel='1'  inherit_from_input=off  hdl_port=on  block_type=gatewayin
  Out1 : Outport
  wires:
  sim_only_reset/1 -> Out1/1
  Pulse Generator/1 -> Add/1
  Pulse Generator1/1 -> Add/2
  Add/1 -> sim_only_reset/1

== system wlan_phy_rx_pmd/Sync & Antenna Sel/Antenna Selection ==
  Pkt Det : Inport
  Rx I Vec : Inport  Port=2
  Rx Q Vec : Inport  Port=3
  IQ Valid : Inport  Port=4
  RSSI Vec : Inport  Port=5
  Reset : Inport  Port=6
  Ant Sel Logic : Subsystem
  Bus Selector1 : BusSelector  OutputSignals=RFA_Q,RFB_Q,RFC_Q,RFD_Q
  Bus Selector2 : BusSelector  OutputSignals=RFA_RSSI,RFB_RSSI,RFC_RSSI,RFD_RSSI
  Bus Selector3 : BusSelector  OutputSignals=RFA_I,RFB_I,RFC_I,RFD_I
  Bus Selector4 : BusSelector  OutputSignals=signal1,signal2,signal3,signal4
  Delay : xbsIndex_r4/Delay  rst=off  infoeditControl=Selection of Reset will increase slice count due to use of real FFs and instead of SRLs  en=off  latency=1  dbl_ovrd=off  reg_retiming=off  block_type=delay
  Gateway Out{,1..2,4..6} (6 alike) : xbsIndex_r4/Gateway Out  inherit_from_input=off  hdl_port=off  timing_constraint=None  locs_specified=off  LOCs={}  UseAsDAC=off  DACChannel='1'  block_type=gatewayout
  Logical : xbsIndex_r4/Logical  logical_function=AND  inputs=2  en=off  latency=0  precision=Full  arith_type=Unsigned  n_bits=16  bin_pt=0  align_bp=on  dbl_ovrd=off  block_type=logical
  Mux{1..4} (4 alike) : xbsIndex_r4/Mux  inputs=4  en=off  latency=0  precision=Full  arith_type=Unsigned  n_bits=16  bin_pt=14  quantization=Truncate  overflow=Wrap  dbl_ovrd=off  block_type=mux
  Posedge : Subsystem
  RF A Gains : Subsystem
  RF B Gains : Subsystem
  RF C Gains : Subsystem
  RF D Gains : Subsystem
  RSSI & Gain Capture : Subsystem
  Register3 : xbsIndex_r4/Register  init=0  rst=off  en=on  dbl_ovrd=off  block_type=register
      settings: xl_area=[1 1 0 0 0 0 0]  block_version=VER_STRING_GOES_HERE
  Sel Div : Scope  NumInputPorts=10
      settings: TimeRange=16708  YMin=0~-1~-1~0~-1~-1~-1~-1~-1~-1  YMax=1~1~1~1~1~1~1~1~1~1  SaveName=ScopeData42
  Rx I : Outport
  " Rx Q" : Outport  Port=2
  " IQ Valid" : Outport  Port=3
  RSSI : Outport  Port=4
  tag scope: ANTENNA_SEL=global
  wires:
  RF A Gains/1(G_vec) -> Gateway Out2/1, Ant Sel Logic/1(G A)
  RF B Gains/1(G_vec) -> Gateway Out4/1, Ant Sel Logic/2(G B)
  Rx Q Vec/1 -> Bus Selector1/1
  Reset/1 -> RF B Gains/2(Rst), RF A Gains/2(Rst), RF C Gains/2(Rst), RF D Gains/2(Rst)
  Logical/1 -> Posedge/1(D)
  Pkt Det/1 -> Logical/1, Gateway Out/1
  [AGC_DONE] -> Logical/2, Gateway Out1/1
  Posedge/1(Q) -> RF A Gains/1(Capt), RF B Gains/1(Capt), RF C Gains/1(Capt), Delay/1, RF D Gains/1(Capt)
  Delay/1 -> Register3/2
  Register3/1 -> [ANTENNA_SEL]
  IQ Valid/1 -> Bus Selector4/1
  Bus Selector4/1 -> Mux3/2  "<signal1>"
  Bus Selector4/2 -> Mux3/3  "<signal2>"
  Mux3/1 -> " IQ Valid"/1
  Gateway Out2/1 -> Sel Div/3  "G A"
  Gateway Out1/1 -> Sel Div/2  "AGC Done"
  Gateway Out/1 -> Sel Div/1  "Pkt Det"
  Gateway Out4/1 -> Sel Div/4  "G B"
  RF C Gains/1(G_vec) -> Ant Sel Logic/3(G C), Gateway Out5/1
  RF D Gains/1(G_vec) -> Ant Sel Logic/4(G D), Gateway Out6/1
  Gateway Out5/1 -> Sel Div/5  "G C"
  Gateway Out6/1 -> Sel Div/6  "G D"
  Bus Selector4/3 -> Mux3/4  "<signal3>"
  Bus Selector4/4 -> Mux3/5  "<signal4>"
  Rx I Vec/1 -> Bus Selector3/1
  Bus Selector3/1 -> Mux1/2  "<RFA_I>"
  Bus Selector3/2 -> Mux1/3  "<RFB_I>"
  Bus Selector3/3 -> Mux1/4  "<RFC_I>"
  Bus Selector3/4 -> Mux1/5  "<RFD_I>"
  Bus Selector1/1 -> Mux2/2  "<RFA_Q>"
  Bus Selector1/2 -> Mux2/3  "<RFB_Q>"
  Bus Selector1/3 -> Mux2/4  "<RFC_Q>"
  Bus Selector1/4 -> Mux2/5  "<RFD_Q>"
  RSSI Vec/1 -> Bus Selector2/1, RSSI & Gain Capture/1(RSSI Vec)
  Bus Selector2/1 -> Mux4/2  "<RFA_RSSI>"
  Bus Selector2/2 -> Mux4/3  "<RFB_RSSI>"
  Bus Selector2/3 -> Mux4/4  "<RFC_RSSI>"
  Bus Selector2/4 -> Mux4/5  "<RFD_RSSI>"
  Mux4/1 -> RSSI/1
  Ant Sel Logic/1(Ant Sel) -> Mux3/1, Mux1/1, Mux2/1, Mux4/1, Register3/1
  Mux2/1 -> " Rx Q"/1
  Mux1/1 -> Rx I/1

== system wlan_phy_rx_pmd/Sync & Antenna Sel/Debug  Outputs ==
  note: Pulse stretch the timeout signal
      | so it's visible on debug outputs
      | and in down-sampled CS ILA
  LTS : Inport
  LTS Timeout : Inport  Port=2
  Det OFDM : Inport  Port=3
  Det DSSS : Inport  Port=4
  Delay : xbsIndex_r4/Delay  rst=off  infoeditControl=Selection of Reset will increase slice count due to use of real FFs and instead of SRLs  en=off  latency=16  dbl_ovrd=off  reg_retiming=off  block_type=delay
  Delay1 : xbsIndex_r4/Delay  rst=off  infoeditControl=Selection of Reset will increase slice count due to use of real FFs and instead of SRLs  en=off  latency=16  dbl_ovrd=off  reg_retiming=off  block_type=delay
  LTS_SYNC : xbsIndex_r4/Gateway Out  inherit_from_input=off  hdl_port=on  timing_constraint=None  locs_specified=off  LOCs={}  UseAsDAC=off  DACChannel='1'  block_type=gatewayout
  Register{2..11} (10 alike) : xbsIndex_r4/Register  init=0  rst=off  en=off  dbl_ovrd=off  block_type=register
  S-R Latch : Subsystem
  S-R Latch1 : Subsystem
  Slice2 : xbsIndex_r4/Slice  nbits=1  boolean_output=on  mode=Upper Bit Location + Width  bit1=0  base1=MSB of Input  bit0=0  base0=LSB of Input  dbl_ovrd=off  block_type=slice
  dbg_PKT_DET_DSSS : xbsIndex_r4/Gateway Out  inherit_from_input=off  hdl_port=on  timing_constraint=None  locs_specified=off  LOCs={}  UseAsDAC=off  DACChannel='1'  block_type=gatewayout
  dbg_PKT_DET_OFDM : xbsIndex_r4/Gateway Out  inherit_from_input=off  hdl_port=on  timing_constraint=None  locs_specified=off  LOCs={}  UseAsDAC=off  DACChannel='1'  block_type=gatewayout
  dbg_lts_timeout : xbsIndex_r4/Gateway Out  inherit_from_input=off  hdl_port=on  timing_constraint=None  locs_specified=off  LOCs={}  UseAsDAC=off  DACChannel='1'  block_type=gatewayout
  tag scope: CS_LTS_Timeout=global, CS_Pktdet_DSSS=global, CS_Pktdet_OFDM=global
  wires:
  S-R Latch/1(Q) -> Delay/1, Register8/1
  Delay/1 -> S-R Latch/2(R)
  Register8/1 -> Register9/1
  Register9/1 -> LTS_SYNC/1
  Slice2/1 -> S-R Latch/1(S)
  LTS Timeout/1 -> S-R Latch1/1(S)
  Register2/1 -> Register3/1
  Register3/1 -> dbg_lts_timeout/1
  Delay1/1 -> S-R Latch1/2(R)
  S-R Latch1/1(Q) -> Register2/1, [CS_LTS_Timeout], Delay1/1
  LTS/1 -> Slice2/1
  Register6/1 -> Register4/1, [CS_Pktdet_OFDM]
  Register7/1 -> dbg_PKT_DET_OFDM/1
  Register4/1 -> Register7/1
  Register10/1 -> Register5/1, [CS_Pktdet_DSSS]
  Register11/1 -> dbg_PKT_DET_DSSS/1
  Register5/1 -> Register11/1
  Det OFDM/1 -> Register6/1
  Det DSSS/1 -> Register10/1

== system wlan_phy_rx_pmd/Sync & Antenna Sel/LTS Correlation ==
  rx_I : Inport
  rx_Q : Inport  Port=2
  rx_IQ_valid : Inport  Port=3
  RSSI : Inport  Port=4
  En : Inport  Port=5
  Reset : Inport  Port=6
  Bus Creator : BusCreator  Inputs=2
  Bus Creator1 : BusCreator  Inputs=2
  Concat : xbsIndex_r4/Concat  num_inputs=2  dbl_ovrd=off  block_type=concat
  Correlator : Subsystem
  Gateway Out{1..4,6..10} (9 alike) : xbsIndex_r4/Gateway Out  inherit_from_input=off  hdl_port=off  timing_constraint=None  locs_specified=off  LOCs={}  UseAsDAC=off  DACChannel='1'  block_type=gatewayout
  Inverter : xbsIndex_r4/Inverter  en=off  latency=0  dbl_ovrd=off  block_type=inv
      settings: xl_area=[0 0 0 0 0 0 0]  block_version=VER_STRING_GOES_HERE
  Inverter1 : xbsIndex_r4/Inverter  en=off  latency=0  dbl_ovrd=off  block_type=inv
      settings: xl_area=[0 0 0 0 0 0 0]  block_version=VER_STRING_GOES_HERE
  LTS Corr : Scope  NumInputPorts=8
      settings: TimeRange=15000  YMin=-1~-1~0~-1~-1~0~-1~-1  YMax=1~1~15000~1~1~1~1~1  SaveName=ScopeData2
  LTS Corr Timeout : Subsystem
  Logical1 : xbsIndex_r4/Logical  logical_function=AND  inputs=2  en=off  latency=0  precision=Full  arith_type=Unsigned  n_bits=16  bin_pt=0  align_bp=on  dbl_ovrd=off  block_type=logical
  Logical2 : xbsIndex_r4/Logical  logical_function=AND  inputs=3  en=off  latency=0  precision=Full  arith_type=Unsigned  n_bits=16  bin_pt=0  align_bp=on  dbl_ovrd=off  block_type=logical
  Logical3 : xbsIndex_r4/Logical  logical_function=AND  inputs=2  en=off  latency=1  precision=Full  arith_type=Unsigned  n_bits=16  bin_pt=0  align_bp=on  dbl_ovrd=off  block_type=logical
  Posedge3 : Subsystem
  Relational2 : xbsIndex_r4/Relational  mode=a>b  en=off  latency=0  dbl_ovrd=off  block_type=relational
      settings: xl_area=[10 0 0 19 0 0 0]  block_version=VER_STRING_GOES_HERE
  S-R Latch : Subsystem
  S-R Latch1 : Subsystem
  Slice : xbsIndex_r4/Slice  nbits=1  boolean_output=off  mode=Upper Bit Location + Width  bit1=0  base1=MSB of Input  bit0=0  base0=LSB of Input  dbl_ovrd=off  block_type=slice
      settings: infoedit="Extracts a given range of bits from each input sample and presents it at the output.  The output type is ordinarily unsigned with binary point at zero, but can be Boolean when the slice is one bit wide.<P><P>Hardware notes: In hardware this block costs nothing."  block_version=10.1.3
  Slice1 : xbsIndex_r4/Slice  nbits=1  boolean_output=off  mode=Upper Bit Location + Width  bit1=0  base1=MSB of Input  bit0=0  base0=LSB of Input  dbl_ovrd=off  block_type=slice
      settings: infoedit="Extracts a given range of bits from each input sample and presents it at the output.  The output type is ordinarily unsigned with binary point at zero, but can be Boolean when the slice is one bit wide.<P><P>Hardware notes: In hardware this block costs nothing."  block_version=10.1.3
  Threshold Select : Subsystem
  Det : Outport
  Timeout : Outport  Port=2
  tag scope: CS_LTS_Det_1=global, CS_LTS_Det_2=global
  wires:
  Gateway Out2/1 -> LTS Corr/2
  Gateway Out4/1 -> LTS Corr/1
  Logical1/1 -> LTS Corr Timeout/3(LTS 1), Concat/2, [CS_LTS_Det_1], Gateway Out8/1
  Inverter/1 -> Logical1/2
  Reset/1 -> S-R Latch/2(R), S-R Latch1/2(R), Gateway Out10/1
  Logical2/1 -> S-R Latch1/1(S), LTS Corr Timeout/4(LTS 2), Concat/1, [CS_LTS_Det_2], Gateway Out9/1
  S-R Latch/1(Q) -> Inverter/1, Logical2/3
  Gateway Out1/1 -> LTS Corr/3
  Gateway Out3/1 -> LTS Corr/4
  rx_I/1 -> Slice/1, Gateway Out4/1
  rx_Q/1 -> Slice1/1, Gateway Out2/1
  Correlator/1(R) -> Relational2/1, Gateway Out1/1
  Gateway Out7/1 -> LTS Corr/7
  Posedge3/1(Q) -> Logical1/1, Logical2/2, S-R Latch/1(S), Gateway Out3/1
  Logical3/1 -> Posedge3/1(D)
  Relational2/1 -> Logical3/2
  En/1 -> LTS Corr Timeout/1(Pkt Det), Logical3/1, Gateway Out6/1, Threshold Select/1(Pkt Det)
  S-R Latch1/1(Q) -> Inverter1/1
  Inverter1/1 -> Logical2/1
  LTS Corr Timeout/1(Timeout) -> Timeout/1, Gateway Out7/1
  Slice/1 -> Correlator/1(Rx I)
  Slice1/1 -> Correlator/2(Rx Q)
  rx_IQ_valid/1 -> LTS Corr Timeout/2(IQ Valid), Correlator/3(rx_IQ_valid)
  Bus Creator/1 -> LTS Corr/5
  Bus Creator1/1 -> LTS Corr/6
  Gateway Out8/1 -> Bus Creator/1
  Gateway Out9/1 -> Bus Creator/2
  Gateway Out6/1 -> Bus Creator1/1
  Gateway Out10/1 -> Bus Creator1/2
  Threshold Select/1(Corr Thresh) -> Relational2/2
  RSSI/1 -> Threshold Select/2(RSSI)
  Concat/1 -> Det/1

== system wlan_phy_rx_pmd/Sync & Antenna Sel/PHY CCA ==
  LTS Sync : Inport
  LTS Timeout : Inport  Port=2
  IQ Valid : Inport  Port=3
  Pkt Det : Inport  Port=4
  Avg RSSI Vec : Inport  Port=5
  Reset : Inport  Port=6
  Bus Selector1 : BusSelector  OutputSignals=RFA_RSSI,RFB_RSSI,RFC_RSSI,RFD_RSSI
  Gateway Out{1..2,4..5,8} (5 alike) : xbsIndex_r4/Gateway Out  inherit_from_input=off  hdl_port=off  timing_constraint=None  locs_specified=off  LOCs={}  UseAsDAC=off  DACChannel='1'  block_type=gatewayout
  Logical1 : xbsIndex_r4/Logical  logical_function=OR  inputs=2  en=off  latency=0  precision=Full  arith_type=Unsigned  n_bits=16  bin_pt=0  align_bp=on  dbl_ovrd=off  block_type=logical
  Logical2 : xbsIndex_r4/Logical  logical_function=OR  inputs=3  en=off  latency=0  precision=Full  arith_type=Unsigned  n_bits=16  bin_pt=0  align_bp=on  dbl_ovrd=off  block_type=logical
  Logical3 : xbsIndex_r4/Logical  logical_function=AND  inputs=2  en=off  latency=0  precision=Full  arith_type=Unsigned  n_bits=16  bin_pt=0  align_bp=on  dbl_ovrd=off  block_type=logical
  PHY CCA : Scope  NumInputPorts=7
      settings: TimeRange=10000  YMin=-100~-100~-100~-70~0~0~-0.25  YMax=100~75~75~80~1~60000~0.3  SaveName=ScopeData31
  PHY CCA Logic : Subsystem
  Rate*Length BUSY : Subsystem
  Relational{,1..3} (4 alike) : xbsIndex_r4/Relational  mode=a>b  en=off  latency=0  dbl_ovrd=off  block_type=relational
  S-R Latch2 : Subsystem
  Slice2 : xbsIndex_r4/Slice  nbits=1  boolean_output=on  mode=Upper Bit Location + Width  bit1=0  base1=MSB of Input  bit0=0  base0=LSB of Input  dbl_ovrd=off  block_type=slice
  tag scope: RX_CCA_BUSY=global
  wires:
  [regRx_RSSI_CS_THRESH] -> Relational/2, Relational1/2, Relational2/2, Relational3/2
  Bus Selector1/1 -> Relational/1, Gateway Out4/1  "<RFA_RSSI>"
  Logical2/1 -> Gateway Out8/1, [RX_CCA_BUSY]  "CCA_BUSY"
  Pkt Det/1 -> S-R Latch2/1(S)
  Logical1/1 -> S-R Latch2/2(R)
  Reset/1 -> Logical1/1
  LTS Timeout/1 -> Logical1/2
  S-R Latch2/1(Q) -> Logical2/3, Gateway Out5/1, Logical3/2
  IQ Valid/1 -> Rate*Length BUSY/1(IQ Valid)
  Logical3/1 -> Rate*Length BUSY/2(Start), Gateway Out1/1
  Rate*Length BUSY/1(PHY Rx Active) -> Logical2/1, Gateway Out2/1
  Gateway Out4/1 -> PHY CCA/1
  Gateway Out1/1 -> PHY CCA/2
  Gateway Out2/1 -> PHY CCA/3
  Gateway Out5/1 -> PHY CCA/5
  Gateway Out8/1 -> PHY CCA/6
  LTS Sync/1 -> Slice2/1
  Avg RSSI Vec/1 -> Bus Selector1/1
  Slice2/1 -> Logical3/1
  Relational/1 -> PHY CCA Logic/1(Det A)
  Relational1/1 -> PHY CCA Logic/2(Det B)
  Bus Selector1/2 -> Relational1/1  "<RFB_RSSI>"
  PHY CCA Logic/1(BUSY) -> Logical2/2
  Bus Selector1/3 -> Relational2/1  "<RFC_RSSI>"
  Bus Selector1/4 -> Relational3/1  "<RFD_RSSI>"
  Relational2/1 -> PHY CCA Logic/3(Det C)
  Relational3/1 -> PHY CCA Logic/4(Det D)
